simulink model "siento_training"  (Simulink 7.2)
settings: Solver=ode45  StartTime=0.0  StopTime=20.0  RelTol=1e-3
undefined symbols (not defined in the file or in its folder): Channel1RTinput, emgGen, fdesignblkfcn, gcbh, scGen
libraries: dspsigops x743, dspbuff3 x3, dspsrcs4 x3
(part 5 of 5)

== matlab function Embedded MATLAB Function1 ==
  data: data input double; featemg output double
function featemg = aubt_proxy_emg(data)
% This block supports the Embedded MATLAB subset.
% See the help menu for details. 
hz=100;
if(min(data)==max(data))
    featvec=rand(1,21);
else
    featvec=zeros(1,21);
    featvec=feval('aubt_extractFeatEMG',data,hz);
end

featemg=featvec';

== matlab function Embedded MATLAB Function ==
  data: data input double; featecg output double
function featecg  = aubt_proxy_ecg(data)
% This block supports the Embedded MATLAB subset.
% See the help menu for details. 
hz=100;
% if(min(data)==max(data))
%     featvec=rand(1,84);
%     %featvec=feval('aubt_extractFeatECG',data,hz)
%     %testing setup
%     %featvec=[sum(data);rand(3,1)];
% else
%     featvec=rand(1,84);
%     %featvec=feval('aubt_extractFeatECG',data,hz);
% end
% featecg=featvec';
featvec=rand(1,84);
featecg=featvec';

== matlab function Embedded MATLAB Function2 ==
  data: data input double; featsc output double
function featsc = aubt_proxy_sc(data)
% This block supports the Embedded MATLAB subset.
% See the help menu for details. 
hz=100;

if(min(data)==max(data))
    featvec=rand(1,21);
else
    featvec=zeros(1,21);
    featvec=feval('aubt_extractFeatSC',data,hz);
end

featsc=featvec';

== matlab function Embedded MATLAB Function3 ==
  data: sam input double; data input double
function   SaveAndFisherProject(sam,data)
% This block supports the Embedded MATLAB subset.
% See the help menu for details. 
data2=data';
sam2=sam';
feval('store',sam2,data2);
feval('fisher_simulink',data2,sam2);
